simulink model "pfb_fft_vacc_cfloat_fft"  (R2016b) (continued)
== system pfb_fft_vacc_cfloat_fft/pfb/fft_wideband_real/fft_biplex_real_4x/biplex_core == (continued)
      | end
      mask Callback use_embedded:
      | % make mutually exclusive with use_hdl.
      | 
      | use_hdl = get_param(gcb, 'use_embedded');
      | if strcmp(use_hdl, 'on'),
      |     set_param_state(gcb, 'use_hdl', 'off');
      | else
      |     set_param_state(gcb, 'use_hdl', 'on');
      | end
      mask Callback hardcode_shifts:
      | hardcode_shifts = get_param(gcb, 'hardcode_shifts');
      | set_param_state(gcb, 'downshift', hardcode_shifts);
      mask Callback dsp48_adders: fft_callback_dsp48_adders(gcb);
  fft_stage_3 : Subsystem  AncestorBlock=casper_library_ffts/fft_stage_n  mask(fft_stage_n){n_inputs=4, FFTSize=10, FFTStage=3, input_bit_width=18, bin_pt_in=9, coeff_bit_width=18, async=on, add_latency=2, mult_latency=3, bram_latency=4, conv_latency=4, quantization=Round  (unbiased: +/- Inf), overflow=Wrap, delays_bram=off, coeffs_bit_limit=13, coeff_sharing=on, coeff_decimation=on, max_fanout=1, use_hdl=on, use_embedded=off, bitgrowth=off, hardcode_shifts=off, downshift=off, dsp48_adders=off}
      mask prompts: n_inputs "Number inputs"; FFTSize "Size of FFT: (2^? pts)"; FFTStage "Stage of FFT:"; input_bit_width "Input Bit Width"; bin_pt_in "Input binary point"; coeff_bit_width "Coefficient Bit Width"; async "Asynchronous operation"; add_latency "Add Latency"; mult_latency "Mult Latency"; bram_latency "BRAM Latency"; conv_latency "Convert latency"; quantization "Quantization Behavior"; overflow "Overflow Behavior"; delays_bram "Implement Delays in BRAM"; coeffs_bit_limit "Number bits above which to store stage's coefficients in BRAM (2^? bits)"; coeff_sharing "BRAM sharing in coeff storage"; coeff_decimation "Store a fraction of coeff factors where useful"; max_fanout "Maximum fanout"; use_hdl "Use behavioural HDL for mutlipliers"; use_embedded "Use embedded multipliers"; bitgrowth "Bit growth instead of shifting"; hardcode_shifts "Hardcode shift schedule"; dsp48_adders "Use DSP48s for adders"
      mask help: FFT internal block.
      mask init: same as pfb_fft_vacc_cfloat_fft/pfb/fft_wideband_real/fft_biplex_real_4x/biplex_core/fft_stage_1
      settings: AttributesFormatString=|
      | [3/10]
      | delays in slices
      | dynamic shifting
      mask TypeOptions quantization: Truncate | Round  (unbiased: +/- Inf) | Round  (unbiased: Even Values)
      mask TypeOptions overflow: Wrap | Saturate | Error
      mask Callback use_hdl:
      | % make mutually exclusive with use_embedded.
      | 
      | use_hdl = get_param(gcb, 'use_hdl');
      | if strcmp(use_hdl, 'on'),
      |     set_param_state(gcb, 'use_embedded', 'off');
      | else
      |     set_param_state(gcb, 'use_embedded', 'on');
      | end
      mask Callback use_embedded:
      | % make mutually exclusive with use_hdl.
      | 
      | use_hdl = get_param(gcb, 'use_embedded');
      | if strcmp(use_hdl, 'on'),
      |     set_param_state(gcb, 'use_hdl', 'off');
      | else
      |     set_param_state(gcb, 'use_hdl', 'on');
      | end
      mask Callback hardcode_shifts:
      | hardcode_shifts = get_param(gcb, 'hardcode_shifts');
      | set_param_state(gcb, 'downshift', hardcode_shifts);
      mask Callback dsp48_adders: fft_callback_dsp48_adders(gcb);
  fft_stage_4 : Subsystem  AncestorBlock=casper_library_ffts/fft_stage_n  mask(fft_stage_n){n_inputs=4, FFTSize=10, FFTStage=4, input_bit_width=18, bin_pt_in=9, coeff_bit_width=18, async=on, add_latency=2, mult_latency=3, bram_latency=4, conv_latency=4, quantization=Round  (unbiased: +/- Inf), overflow=Wrap, delays_bram=off, coeffs_bit_limit=13, coeff_sharing=on, coeff_decimation=on, max_fanout=1, use_hdl=on, use_embedded=off, bitgrowth=off, hardcode_shifts=off, downshift=off, dsp48_adders=off}
      mask prompts: n_inputs "Number inputs"; FFTSize "Size of FFT: (2^? pts)"; FFTStage "Stage of FFT:"; input_bit_width "Input Bit Width"; bin_pt_in "Input binary point"; coeff_bit_width "Coefficient Bit Width"; async "Asynchronous operation"; add_latency "Add Latency"; mult_latency "Mult Latency"; bram_latency "BRAM Latency"; conv_latency "Convert latency"; quantization "Quantization Behavior"; overflow "Overflow Behavior"; delays_bram "Implement Delays in BRAM"; coeffs_bit_limit "Number bits above which to store stage's coefficients in BRAM (2^? bits)"; coeff_sharing "BRAM sharing in coeff storage"; coeff_decimation "Store a fraction of coeff factors where useful"; max_fanout "Maximum fanout"; use_hdl "Use behavioural HDL for mutlipliers"; use_embedded "Use embedded multipliers"; bitgrowth "Bit growth instead of shifting"; hardcode_shifts "Hardcode shift schedule"; dsp48_adders "Use DSP48s for adders"
      mask help: FFT internal block.
      mask init: same as pfb_fft_vacc_cfloat_fft/pfb/fft_wideband_real/fft_biplex_real_4x/biplex_core/fft_stage_1
      settings: AttributesFormatString=|
      | [4/10]
      | delays in slices
      | dynamic shifting
      mask TypeOptions quantization: Truncate | Round  (unbiased: +/- Inf) | Round  (unbiased: Even Values)
      mask TypeOptions overflow: Wrap | Saturate | Error
      mask Callback use_hdl:
      | % make mutually exclusive with use_embedded.
      | 
      | use_hdl = get_param(gcb, 'use_hdl');
      | if strcmp(use_hdl, 'on'),
      |     set_param_state(gcb, 'use_embedded', 'off');
      | else
      |     set_param_state(gcb, 'use_embedded', 'on');
      | end
      mask Callback use_embedded:
      | % make mutually exclusive with use_hdl.
      | 
      | use_hdl = get_param(gcb, 'use_embedded');
      | if strcmp(use_hdl, 'on'),
      |     set_param_state(gcb, 'use_hdl', 'off');
      | else
      |     set_param_state(gcb, 'use_hdl', 'on');
      | end
      mask Callback hardcode_shifts:
      | hardcode_shifts = get_param(gcb, 'hardcode_shifts');
      | set_param_state(gcb, 'downshift', hardcode_shifts);
      mask Callback dsp48_adders: fft_callback_dsp48_adders(gcb);
  fft_stage_5 : Subsystem  AncestorBlock=casper_library_ffts/fft_stage_n  mask(fft_stage_n){n_inputs=4, FFTSize=10, FFTStage=5, input_bit_width=18, bin_pt_in=9, coeff_bit_width=18, async=on, add_latency=2, mult_latency=3, bram_latency=4, conv_latency=4, quantization=Round  (unbiased: +/- Inf), overflow=Wrap, delays_bram=off, coeffs_bit_limit=13, coeff_sharing=on, coeff_decimation=on, max_fanout=1, use_hdl=on, use_embedded=off, bitgrowth=off, hardcode_shifts=off, downshift=off, dsp48_adders=off}
      mask prompts: n_inputs "Number inputs"; FFTSize "Size of FFT: (2^? pts)"; FFTStage "Stage of FFT:"; input_bit_width "Input Bit Width"; bin_pt_in "Input binary point"; coeff_bit_width "Coefficient Bit Width"; async "Asynchronous operation"; add_latency "Add Latency"; mult_latency "Mult Latency"; bram_latency "BRAM Latency"; conv_latency "Convert latency"; quantization "Quantization Behavior"; overflow "Overflow Behavior"; delays_bram "Implement Delays in BRAM"; coeffs_bit_limit "Number bits above which to store stage's coefficients in BRAM (2^? bits)"; coeff_sharing "BRAM sharing in coeff storage"; coeff_decimation "Store a fraction of coeff factors where useful"; max_fanout "Maximum fanout"; use_hdl "Use behavioural HDL for mutlipliers"; use_embedded "Use embedded multipliers"; bitgrowth "Bit growth instead of shifting"; hardcode_shifts "Hardcode shift schedule"; dsp48_adders "Use DSP48s for adders"
      mask help: FFT internal block.
      mask init: same as pfb_fft_vacc_cfloat_fft/pfb/fft_wideband_real/fft_biplex_real_4x/biplex_core/fft_stage_1
      settings: AttributesFormatString=|
      | [5/10]
      | delays in slices
      | dynamic shifting
      mask TypeOptions quantization: Truncate | Round  (unbiased: +/- Inf) | Round  (unbiased: Even Values)
      mask TypeOptions overflow: Wrap | Saturate | Error
      mask Callback use_hdl:
      | % make mutually exclusive with use_embedded.
      | 
      | use_hdl = get_param(gcb, 'use_hdl');
      | if strcmp(use_hdl, 'on'),
      |     set_param_state(gcb, 'use_embedded', 'off');
      | else
      |     set_param_state(gcb, 'use_embedded', 'on');
      | end
      mask Callback use_embedded:
      | % make mutually exclusive with use_hdl.
      | 
      | use_hdl = get_param(gcb, 'use_embedded');
      | if strcmp(use_hdl, 'on'),
      |     set_param_state(gcb, 'use_hdl', 'off');
      | else
      |     set_param_state(gcb, 'use_hdl', 'on');
      | end
      mask Callback hardcode_shifts:
      | hardcode_shifts = get_param(gcb, 'hardcode_shifts');
      | set_param_state(gcb, 'downshift', hardcode_shifts);
      mask Callback dsp48_adders: fft_callback_dsp48_adders(gcb);
  fft_stage_6 : Subsystem  AncestorBlock=casper_library_ffts/fft_stage_n  mask(fft_stage_n){n_inputs=4, FFTSize=10, FFTStage=6, input_bit_width=18, bin_pt_in=9, coeff_bit_width=18, async=on, add_latency=2, mult_latency=3, bram_latency=4, conv_latency=4, quantization=Round  (unbiased: +/- Inf), overflow=Wrap, delays_bram=off, coeffs_bit_limit=13, coeff_sharing=on, coeff_decimation=on, max_fanout=1, use_hdl=on, use_embedded=off, bitgrowth=off, hardcode_shifts=off, downshift=off, dsp48_adders=off}
      mask prompts: n_inputs "Number inputs"; FFTSize "Size of FFT: (2^? pts)"; FFTStage "Stage of FFT:"; input_bit_width "Input Bit Width"; bin_pt_in "Input binary point"; coeff_bit_width "Coefficient Bit Width"; async "Asynchronous operation"; add_latency "Add Latency"; mult_latency "Mult Latency"; bram_latency "BRAM Latency"; conv_latency "Convert latency"; quantization "Quantization Behavior"; overflow "Overflow Behavior"; delays_bram "Implement Delays in BRAM"; coeffs_bit_limit "Number bits above which to store stage's coefficients in BRAM (2^? bits)"; coeff_sharing "BRAM sharing in coeff storage"; coeff_decimation "Store a fraction of coeff factors where useful"; max_fanout "Maximum fanout"; use_hdl "Use behavioural HDL for mutlipliers"; use_embedded "Use embedded multipliers"; bitgrowth "Bit growth instead of shifting"; hardcode_shifts "Hardcode shift schedule"; dsp48_adders "Use DSP48s for adders"
      mask help: FFT internal block.
      mask init: same as pfb_fft_vacc_cfloat_fft/pfb/fft_wideband_real/fft_biplex_real_4x/biplex_core/fft_stage_1
      settings: AttributesFormatString=|
      | [6/10]
      | delays in slices
      | dynamic shifting
      mask TypeOptions quantization: Truncate | Round  (unbiased: +/- Inf) | Round  (unbiased: Even Values)
      mask TypeOptions overflow: Wrap | Saturate | Error
      mask Callback use_hdl:
      | % make mutually exclusive with use_embedded.
      | 
      | use_hdl = get_param(gcb, 'use_hdl');
      | if strcmp(use_hdl, 'on'),
      |     set_param_state(gcb, 'use_embedded', 'off');
      | else
      |     set_param_state(gcb, 'use_embedded', 'on');
      | end
      mask Callback use_embedded:
      | % make mutually exclusive with use_hdl.
      | 
      | use_hdl = get_param(gcb, 'use_embedded');
      | if strcmp(use_hdl, 'on'),
      |     set_param_state(gcb, 'use_hdl', 'off');
      | else
      |     set_param_state(gcb, 'use_hdl', 'on');
      | end
      mask Callback hardcode_shifts:
      | hardcode_shifts = get_param(gcb, 'hardcode_shifts');
      | set_param_state(gcb, 'downshift', hardcode_shifts);
      mask Callback dsp48_adders: fft_callback_dsp48_adders(gcb);
  fft_stage_7 : Subsystem  AncestorBlock=casper_library_ffts/fft_stage_n  mask(fft_stage_n){n_inputs=4, FFTSize=10, FFTStage=7, input_bit_width=18, bin_pt_in=9, coeff_bit_width=18, async=on, add_latency=2, mult_latency=3, bram_latency=4, conv_latency=4, quantization=Round  (unbiased: +/- Inf), overflow=Wrap, delays_bram=off, coeffs_bit_limit=13, coeff_sharing=on, coeff_decimation=on, max_fanout=1, use_hdl=on, use_embedded=off, bitgrowth=off, hardcode_shifts=off, downshift=off, dsp48_adders=off}
      mask prompts: n_inputs "Number inputs"; FFTSize "Size of FFT: (2^? pts)"; FFTStage "Stage of FFT:"; input_bit_width "Input Bit Width"; bin_pt_in "Input binary point"; coeff_bit_width "Coefficient Bit Width"; async "Asynchronous operation"; add_latency "Add Latency"; mult_latency "Mult Latency"; bram_latency "BRAM Latency"; conv_latency "Convert latency"; quantization "Quantization Behavior"; overflow "Overflow Behavior"; delays_bram "Implement Delays in BRAM"; coeffs_bit_limit "Number bits above which to store stage's coefficients in BRAM (2^? bits)"; coeff_sharing "BRAM sharing in coeff storage"; coeff_decimation "Store a fraction of coeff factors where useful"; max_fanout "Maximum fanout"; use_hdl "Use behavioural HDL for mutlipliers"; use_embedded "Use embedded multipliers"; bitgrowth "Bit growth instead of shifting"; hardcode_shifts "Hardcode shift schedule"; dsp48_adders "Use DSP48s for adders"
      mask help: FFT internal block.
      mask init: same as pfb_fft_vacc_cfloat_fft/pfb/fft_wideband_real/fft_biplex_real_4x/biplex_core/fft_stage_1
      settings: AttributesFormatString=|
      | [7/10]
      | delays in slices
      | dynamic shifting
      mask TypeOptions quantization: Truncate | Round  (unbiased: +/- Inf) | Round  (unbiased: Even Values)
      mask TypeOptions overflow: Wrap | Saturate | Error
      mask Callback use_hdl:
      | % make mutually exclusive with use_embedded.
      | 
      | use_hdl = get_param(gcb, 'use_hdl');
      | if strcmp(use_hdl, 'on'),
      |     set_param_state(gcb, 'use_embedded', 'off');
      | else
      |     set_param_state(gcb, 'use_embedded', 'on');
      | end
      mask Callback use_embedded:
      | % make mutually exclusive with use_hdl.
      | 
      | use_hdl = get_param(gcb, 'use_embedded');
      | if strcmp(use_hdl, 'on'),
      |     set_param_state(gcb, 'use_hdl', 'off');
      | else
      |     set_param_state(gcb, 'use_hdl', 'on');
      | end
      mask Callback hardcode_shifts:
      | hardcode_shifts = get_param(gcb, 'hardcode_shifts');
      | set_param_state(gcb, 'downshift', hardcode_shifts);
      mask Callback dsp48_adders: fft_callback_dsp48_adders(gcb);
  fft_stage_8 : Subsystem  AncestorBlock=casper_library_ffts/fft_stage_n  mask(fft_stage_n){n_inputs=4, FFTSize=10, FFTStage=8, input_bit_width=18, bin_pt_in=9, coeff_bit_width=18, async=on, add_latency=2, mult_latency=3, bram_latency=4, conv_latency=4, quantization=Round  (unbiased: +/- Inf), overflow=Wrap, delays_bram=off, coeffs_bit_limit=13, coeff_sharing=on, coeff_decimation=on, max_fanout=1, use_hdl=on, use_embedded=off, bitgrowth=off, hardcode_shifts=off, downshift=off, dsp48_adders=off}
      mask prompts: n_inputs "Number inputs"; FFTSize "Size of FFT: (2^? pts)"; FFTStage "Stage of FFT:"; input_bit_width "Input Bit Width"; bin_pt_in "Input binary point"; coeff_bit_width "Coefficient Bit Width"; async "Asynchronous operation"; add_latency "Add Latency"; mult_latency "Mult Latency"; bram_latency "BRAM Latency"; conv_latency "Convert latency"; quantization "Quantization Behavior"; overflow "Overflow Behavior"; delays_bram "Implement Delays in BRAM"; coeffs_bit_limit "Number bits above which to store stage's coefficients in BRAM (2^? bits)"; coeff_sharing "BRAM sharing in coeff storage"; coeff_decimation "Store a fraction of coeff factors where useful"; max_fanout "Maximum fanout"; use_hdl "Use behavioural HDL for mutlipliers"; use_embedded "Use embedded multipliers"; bitgrowth "Bit growth instead of shifting"; hardcode_shifts "Hardcode shift schedule"; dsp48_adders "Use DSP48s for adders"
      mask help: FFT internal block.
      mask init: same as pfb_fft_vacc_cfloat_fft/pfb/fft_wideband_real/fft_biplex_real_4x/biplex_core/fft_stage_1
      settings: AttributesFormatString=|
      | [8/10]
      | delays in slices
      | dynamic shifting
      mask TypeOptions quantization: Truncate | Round  (unbiased: +/- Inf) | Round  (unbiased: Even Values)
      mask TypeOptions overflow: Wrap | Saturate | Error
      mask Callback use_hdl:
      | % make mutually exclusive with use_embedded.
      | 
      | use_hdl = get_param(gcb, 'use_hdl');
      | if strcmp(use_hdl, 'on'),
      |     set_param_state(gcb, 'use_embedded', 'off');
      | else
      |     set_param_state(gcb, 'use_embedded', 'on');
      | end
      mask Callback use_embedded:
      | % make mutually exclusive with use_hdl.
      | 
      | use_hdl = get_param(gcb, 'use_embedded');
      | if strcmp(use_hdl, 'on'),
      |     set_param_state(gcb, 'use_hdl', 'off');
      | else
      |     set_param_state(gcb, 'use_hdl', 'on');
      | end
      mask Callback hardcode_shifts:
      | hardcode_shifts = get_param(gcb, 'hardcode_shifts');
      | set_param_state(gcb, 'downshift', hardcode_shifts);
      mask Callback dsp48_adders: fft_callback_dsp48_adders(gcb);
  fft_stage_9 : Subsystem  AncestorBlock=casper_library_ffts/fft_stage_n  mask(fft_stage_n){n_inputs=4, FFTSize=10, FFTStage=9, input_bit_width=18, bin_pt_in=9, coeff_bit_width=18, async=on, add_latency=2, mult_latency=3, bram_latency=4, conv_latency=4, quantization=Round  (unbiased: +/- Inf), overflow=Wrap, delays_bram=off, coeffs_bit_limit=13, coeff_sharing=on, coeff_decimation=on, max_fanout=1, use_hdl=on, use_embedded=off, bitgrowth=off, hardcode_shifts=off, downshift=off, dsp48_adders=off}
      mask prompts: n_inputs "Number inputs"; FFTSize "Size of FFT: (2^? pts)"; FFTStage "Stage of FFT:"; input_bit_width "Input Bit Width"; bin_pt_in "Input binary point"; coeff_bit_width "Coefficient Bit Width"; async "Asynchronous operation"; add_latency "Add Latency"; mult_latency "Mult Latency"; bram_latency "BRAM Latency"; conv_latency "Convert latency"; quantization "Quantization Behavior"; overflow "Overflow Behavior"; delays_bram "Implement Delays in BRAM"; coeffs_bit_limit "Number bits above which to store stage's coefficients in BRAM (2^? bits)"; coeff_sharing "BRAM sharing in coeff storage"; coeff_decimation "Store a fraction of coeff factors where useful"; max_fanout "Maximum fanout"; use_hdl "Use behavioural HDL for mutlipliers"; use_embedded "Use embedded multipliers"; bitgrowth "Bit growth instead of shifting"; hardcode_shifts "Hardcode shift schedule"; dsp48_adders "Use DSP48s for adders"
      mask help: FFT internal block.
      mask init: same as pfb_fft_vacc_cfloat_fft/pfb/fft_wideband_real/fft_biplex_real_4x/biplex_core/fft_stage_1
      settings: AttributesFormatString=|
      | [9/10]
      | delays in slices
      | dynamic shifting
      mask TypeOptions quantization: Truncate | Round  (unbiased: +/- Inf) | Round  (unbiased: Even Values)
      mask TypeOptions overflow: Wrap | Saturate | Error
      mask Callback use_hdl:
      | % make mutually exclusive with use_embedded.
      | 
      | use_hdl = get_param(gcb, 'use_hdl');
      | if strcmp(use_hdl, 'on'),
      |     set_param_state(gcb, 'use_embedded', 'off');
      | else
      |     set_param_state(gcb, 'use_embedded', 'on');
      | end
      mask Callback use_embedded:
      | % make mutually exclusive with use_hdl.
      | 
      | use_hdl = get_param(gcb, 'use_embedded');
      | if strcmp(use_hdl, 'on'),
      |     set_param_state(gcb, 'use_hdl', 'off');
      | else
      |     set_param_state(gcb, 'use_hdl', 'on');
      | end
      mask Callback hardcode_shifts:
      | hardcode_shifts = get_param(gcb, 'hardcode_shifts');
      | set_param_state(gcb, 'downshift', hardcode_shifts);
      mask Callback dsp48_adders: fft_callback_dsp48_adders(gcb);
  sync_out : Outport
  out1 : Outport  Port=2
  out2 : Outport  Port=3
  of : Outport  Port=4
  dvalid : Outport  Port=5
  wires:
  pol1/1 -> fft_stage_1/1(in1)
  pol2/1 -> fft_stage_1/2(in2)
  Constant/1 -> fft_stage_1/3(of_in)
  sync/1 -> fft_stage_1/4(sync)
  fft_stage_10/1(out1) -> bus_expand10/1(bus_in), out1/1
  fft_stage_10/2(out2) -> out2/1
  fft_stage_10/3(of) -> of/1
  fft_stage_10/4(sync_out) -> sync_out/1
  en/1 -> fft_stage_1/6(en)
  fft_stage_10/5(dvalid) -> dvalid/1
  fft_stage_1/2(out2) -> fft_stage_2/2(in2)
  fft_stage_1/3(of) -> fft_stage_2/3(of_in)
  fft_stage_1/4(sync_out) -> fft_stage_2/4(sync)
  shift/1 -> fft_stage_2/5(shift), fft_stage_10/5(shift), fft_stage_9/5(shift), fft_stage_8/5(shift), fft_stage_7/5(shift), fft_stage_6/5(shift), fft_stage_5/5(shift), fft_stage_4/5(shift), fft_stage_3/5(shift), fft_stage_1/5(shift)
  fft_stage_1/5(dvalid) -> fft_stage_2/6(en)
  fft_stage_2/1(out1) -> bus_expand2/1(bus_in), fft_stage_3/1(in1)
  fft_stage_2/2(out2) -> fft_stage_3/2(in2)
  fft_stage_2/3(of) -> fft_stage_3/3(of_in)
  fft_stage_2/4(sync_out) -> fft_stage_3/4(sync)
  fft_stage_2/5(dvalid) -> fft_stage_3/6(en)
  fft_stage_3/1(out1) -> bus_expand3/1(bus_in), fft_stage_4/1(in1)
  fft_stage_3/3(of) -> fft_stage_4/3(of_in)
  fft_stage_3/4(sync_out) -> fft_stage_4/4(sync)
  fft_stage_3/5(dvalid) -> fft_stage_4/6(en)
  fft_stage_3/2(out2) -> fft_stage_4/2(in2)
  fft_stage_4/1(out1) -> bus_expand4/1(bus_in), fft_stage_5/1(in1)
  fft_stage_4/3(of) -> fft_stage_5/3(of_in)
  fft_stage_4/2(out2) -> fft_stage_5/2(in2)
  fft_stage_4/5(dvalid) -> fft_stage_5/6(en)
  fft_stage_4/4(sync_out) -> fft_stage_5/4(sync)
  fft_stage_5/2(out2) -> fft_stage_6/2(in2)
  fft_stage_5/3(of) -> fft_stage_6/3(of_in)
  fft_stage_5/4(sync_out) -> fft_stage_6/4(sync)
  fft_stage_5/1(out1) -> bus_expand5/1(bus_in), fft_stage_6/1(in1)
  fft_stage_5/5(dvalid) -> fft_stage_6/6(en)
  fft_stage_6/5(dvalid) -> fft_stage_7/6(en)
  fft_stage_6/1(out1) -> bus_expand6/1(bus_in), fft_stage_7/1(in1)
  fft_stage_6/3(of) -> fft_stage_7/3(of_in)
  fft_stage_6/4(sync_out) -> fft_stage_7/4(sync)
  fft_stage_6/2(out2) -> fft_stage_7/2(in2)
  fft_stage_7/4(sync_out) -> fft_stage_8/4(sync)
  fft_stage_7/3(of) -> fft_stage_8/3(of_in)
  fft_stage_7/5(dvalid) -> fft_stage_8/6(en)
  fft_stage_7/1(out1) -> bus_expand7/1(bus_in), fft_stage_8/1(in1)
  fft_stage_7/2(out2) -> fft_stage_8/2(in2)
  fft_stage_8/5(dvalid) -> fft_stage_9/6(en)
  fft_stage_8/4(sync_out) -> fft_stage_9/4(sync)
  fft_stage_8/2(out2) -> fft_stage_9/2(in2)
  fft_stage_8/3(of) -> fft_stage_9/3(of_in)
  fft_stage_8/1(out1) -> bus_expand8/1(bus_in), fft_stage_9/1(in1)
  fft_stage_9/1(out1) -> bus_expand9/1(bus_in), fft_stage_10/1(in1)
  fft_stage_9/5(dvalid) -> fft_stage_10/6(en)
  fft_stage_9/4(sync_out) -> fft_stage_10/4(sync)
  fft_stage_9/2(out2) -> fft_stage_10/2(in2)
  fft_stage_9/3(of) -> fft_stage_10/3(of_in)
  fft_stage_1/1(out1) -> bus_expand1/1(bus_in), fft_stage_2/1(in1)
  Gateway Out6/1 -> To Workspace5/1
  Reinterpret4/1 -> Gateway Out6/1
  bus_expand1/1(msb_out8) -> Reinterpret4/1
  Gateway Out1/1 -> To Workspace1/1
  Reinterpret1/1 -> Gateway Out1/1
  bus_expand1/2(out7) -> Reinterpret1/1
  Gateway Out2/1 -> To Workspace2/1
  Reinterpret2/1 -> Gateway Out2/1
  bus_expand1/3(out6) -> Reinterpret2/1
  Gateway Out3/1 -> To Workspace3/1
  Reinterpret3/1 -> Gateway Out3/1
  bus_expand1/4(out5) -> Reinterpret3/1
  Gateway Out4/1 -> To Workspace4/1
  Reinterpret5/1 -> Gateway Out4/1
  bus_expand1/5(out4) -> Reinterpret5/1
  Gateway Out5/1 -> To Workspace6/1
  Reinterpret6/1 -> Gateway Out5/1
  bus_expand1/6(out3) -> Reinterpret6/1
  Gateway Out7/1 -> To Workspace7/1
  Reinterpret7/1 -> Gateway Out7/1
  bus_expand1/7(out2) -> Reinterpret7/1
  Gateway Out8/1 -> To Workspace8/1
  Reinterpret8/1 -> Gateway Out8/1
  bus_expand1/8(lsb_out1) -> Reinterpret8/1
  Gateway Out14/1 -> To Workspace13/1
  Reinterpret12/1 -> Gateway Out14/1
  bus_expand2/1(msb_out8) -> Reinterpret12/1
  Gateway Out9/1 -> To Workspace9/1
  Reinterpret9/1 -> Gateway Out9/1
  bus_expand2/2(out7) -> Reinterpret9/1
  Gateway Out10/1 -> To Workspace10/1
  Reinterpret10/1 -> Gateway Out10/1
  bus_expand2/3(out6) -> Reinterpret10/1
  Gateway Out11/1 -> To Workspace11/1
  Reinterpret11/1 -> Gateway Out11/1
  bus_expand2/4(out5) -> Reinterpret11/1
  Gateway Out12/1 -> To Workspace12/1
  Reinterpret13/1 -> Gateway Out12/1
  bus_expand2/5(out4) -> Reinterpret13/1
  Gateway Out13/1 -> To Workspace14/1
  Reinterpret14/1 -> Gateway Out13/1
  bus_expand2/6(out3) -> Reinterpret14/1
  Gateway Out15/1 -> To Workspace15/1
  Reinterpret15/1 -> Gateway Out15/1
  bus_expand2/7(out2) -> Reinterpret15/1
  Gateway Out16/1 -> To Workspace16/1
  Reinterpret16/1 -> Gateway Out16/1
  bus_expand2/8(lsb_out1) -> Reinterpret16/1
  Gateway Out21/1 -> To Workspace20/1
  Reinterpret19/1 -> Gateway Out21/1
  bus_expand3/1(msb_out8) -> Reinterpret19/1
  Gateway Out24/1 -> To Workspace24/1
  Reinterpret24/1 -> Gateway Out24/1
  bus_expand3/2(out7) -> Reinterpret24/1
  Gateway Out17/1 -> To Workspace17/1
  Reinterpret17/1 -> Gateway Out17/1
  bus_expand3/3(out6) -> Reinterpret17/1
  Gateway Out18/1 -> To Workspace18/1
  Reinterpret18/1 -> Gateway Out18/1
  bus_expand3/4(out5) -> Reinterpret18/1
  Gateway Out19/1 -> To Workspace19/1
  Reinterpret20/1 -> Gateway Out19/1
  bus_expand3/5(out4) -> Reinterpret20/1
  Gateway Out20/1 -> To Workspace21/1
  Reinterpret21/1 -> Gateway Out20/1
  bus_expand3/6(out3) -> Reinterpret21/1
  Gateway Out22/1 -> To Workspace22/1
  Reinterpret22/1 -> Gateway Out22/1
  bus_expand3/7(out2) -> Reinterpret22/1
  Gateway Out23/1 -> To Workspace23/1
  Reinterpret23/1 -> Gateway Out23/1
  bus_expand3/8(lsb_out1) -> Reinterpret23/1
  Gateway Out29/1 -> To Workspace28/1
  Reinterpret27/1 -> Gateway Out29/1
  bus_expand4/1(msb_out8) -> Reinterpret27/1
  Gateway Out32/1 -> To Workspace32/1
  Reinterpret32/1 -> Gateway Out32/1
  bus_expand4/2(out7) -> Reinterpret32/1
  Gateway Out25/1 -> To Workspace25/1
  Reinterpret25/1 -> Gateway Out25/1
  bus_expand4/3(out6) -> Reinterpret25/1
  Gateway Out26/1 -> To Workspace26/1
  Reinterpret26/1 -> Gateway Out26/1
  bus_expand4/4(out5) -> Reinterpret26/1
  Gateway Out27/1 -> To Workspace27/1
  Reinterpret28/1 -> Gateway Out27/1
  bus_expand4/5(out4) -> Reinterpret28/1
  Gateway Out28/1 -> To Workspace29/1
  Reinterpret29/1 -> Gateway Out28/1
  bus_expand4/6(out3) -> Reinterpret29/1
  Gateway Out30/1 -> To Workspace30/1
  Reinterpret30/1 -> Gateway Out30/1
  bus_expand4/7(out2) -> Reinterpret30/1
  Gateway Out31/1 -> To Workspace31/1
  Reinterpret31/1 -> Gateway Out31/1
  bus_expand4/8(lsb_out1) -> Reinterpret31/1
  Gateway Out37/1 -> To Workspace36/1
  Reinterpret35/1 -> Gateway Out37/1
  bus_expand5/1(msb_out8) -> Reinterpret35/1
  Gateway Out40/1 -> To Workspace40/1
  Reinterpret40/1 -> Gateway Out40/1
  bus_expand5/2(out7) -> Reinterpret40/1
  Gateway Out33/1 -> To Workspace33/1
  Reinterpret33/1 -> Gateway Out33/1
  bus_expand5/3(out6) -> Reinterpret33/1
  Gateway Out34/1 -> To Workspace34/1
  Reinterpret34/1 -> Gateway Out34/1
  bus_expand5/4(out5) -> Reinterpret34/1
  Gateway Out35/1 -> To Workspace35/1
  Reinterpret36/1 -> Gateway Out35/1
  bus_expand5/5(out4) -> Reinterpret36/1
  Gateway Out36/1 -> To Workspace37/1
  Reinterpret37/1 -> Gateway Out36/1
  bus_expand5/6(out3) -> Reinterpret37/1
  Gateway Out38/1 -> To Workspace38/1
  Reinterpret38/1 -> Gateway Out38/1
  bus_expand5/7(out2) -> Reinterpret38/1
  Gateway Out39/1 -> To Workspace39/1
  Reinterpret39/1 -> Gateway Out39/1
  bus_expand5/8(lsb_out1) -> Reinterpret39/1
  Gateway Out45/1 -> To Workspace44/1
  Reinterpret43/1 -> Gateway Out45/1
  bus_expand6/1(msb_out8) -> Reinterpret43/1
  Gateway Out48/1 -> To Workspace48/1
  Reinterpret48/1 -> Gateway Out48/1
  bus_expand6/2(out7) -> Reinterpret48/1
  Gateway Out41/1 -> To Workspace41/1
  Reinterpret41/1 -> Gateway Out41/1
  bus_expand6/3(out6) -> Reinterpret41/1
  Gateway Out42/1 -> To Workspace42/1
  Reinterpret42/1 -> Gateway Out42/1
  bus_expand6/4(out5) -> Reinterpret42/1
  Gateway Out43/1 -> To Workspace43/1
  Reinterpret44/1 -> Gateway Out43/1
  bus_expand6/5(out4) -> Reinterpret44/1
  Gateway Out44/1 -> To Workspace45/1
  Reinterpret45/1 -> Gateway Out44/1
  bus_expand6/6(out3) -> Reinterpret45/1
  Gateway Out46/1 -> To Workspace46/1
  Reinterpret46/1 -> Gateway Out46/1
  bus_expand6/7(out2) -> Reinterpret46/1
  Gateway Out47/1 -> To Workspace47/1
  Reinterpret47/1 -> Gateway Out47/1
  bus_expand6/8(lsb_out1) -> Reinterpret47/1
  Gateway Out53/1 -> To Workspace52/1
  Reinterpret51/1 -> Gateway Out53/1
  bus_expand7/1(msb_out8) -> Reinterpret51/1
  Gateway Out56/1 -> To Workspace56/1
  Reinterpret56/1 -> Gateway Out56/1
  bus_expand7/2(out7) -> Reinterpret56/1
  Gateway Out49/1 -> To Workspace49/1
  Reinterpret49/1 -> Gateway Out49/1
  bus_expand7/3(out6) -> Reinterpret49/1
  Gateway Out50/1 -> To Workspace50/1
  Reinterpret50/1 -> Gateway Out50/1
  bus_expand7/4(out5) -> Reinterpret50/1
  Gateway Out51/1 -> To Workspace51/1
  Reinterpret52/1 -> Gateway Out51/1
  bus_expand7/5(out4) -> Reinterpret52/1
  Gateway Out52/1 -> To Workspace53/1
  Reinterpret53/1 -> Gateway Out52/1
  bus_expand7/6(out3) -> Reinterpret53/1
  Gateway Out54/1 -> To Workspace54/1
  Reinterpret54/1 -> Gateway Out54/1
  bus_expand7/7(out2) -> Reinterpret54/1
  Gateway Out55/1 -> To Workspace55/1
  Reinterpret55/1 -> Gateway Out55/1
  bus_expand7/8(lsb_out1) -> Reinterpret55/1
  Gateway Out61/1 -> To Workspace60/1
  Reinterpret59/1 -> Gateway Out61/1
  bus_expand8/1(msb_out8) -> Reinterpret59/1
  Gateway Out64/1 -> To Workspace64/1
  Reinterpret64/1 -> Gateway Out64/1
  bus_expand8/2(out7) -> Reinterpret64/1
  Gateway Out57/1 -> To Workspace57/1
  Reinterpret57/1 -> Gateway Out57/1
  bus_expand8/3(out6) -> Reinterpret57/1
  Gateway Out58/1 -> To Workspace58/1
  Reinterpret58/1 -> Gateway Out58/1
  bus_expand8/4(out5) -> Reinterpret58/1
  Gateway Out59/1 -> To Workspace59/1
  Reinterpret60/1 -> Gateway Out59/1
  bus_expand8/5(out4) -> Reinterpret60/1
  Gateway Out60/1 -> To Workspace61/1
  Reinterpret61/1 -> Gateway Out60/1
  bus_expand8/6(out3) -> Reinterpret61/1
  Gateway Out62/1 -> To Workspace62/1
  Reinterpret62/1 -> Gateway Out62/1
  bus_expand8/7(out2) -> Reinterpret62/1
  Gateway Out63/1 -> To Workspace63/1
  Reinterpret63/1 -> Gateway Out63/1
  bus_expand8/8(lsb_out1) -> Reinterpret63/1
  Gateway Out69/1 -> To Workspace68/1
  Reinterpret67/1 -> Gateway Out69/1
  bus_expand9/1(msb_out8) -> Reinterpret67/1
  Gateway Out72/1 -> To Workspace72/1
  Reinterpret72/1 -> Gateway Out72/1
  bus_expand9/2(out7) -> Reinterpret72/1
  Gateway Out65/1 -> To Workspace65/1
  Reinterpret65/1 -> Gateway Out65/1
  bus_expand9/3(out6) -> Reinterpret65/1
  Gateway Out66/1 -> To Workspace66/1
  Reinterpret66/1 -> Gateway Out66/1
  bus_expand9/4(out5) -> Reinterpret66/1
  Gateway Out67/1 -> To Workspace67/1
  Reinterpret68/1 -> Gateway Out67/1
  bus_expand9/5(out4) -> Reinterpret68/1
  Gateway Out68/1 -> To Workspace69/1
  Reinterpret69/1 -> Gateway Out68/1
  bus_expand9/6(out3) -> Reinterpret69/1
  Gateway Out70/1 -> To Workspace70/1
  Reinterpret70/1 -> Gateway Out70/1
  bus_expand9/7(out2) -> Reinterpret70/1
  Gateway Out71/1 -> To Workspace71/1
  Reinterpret71/1 -> Gateway Out71/1
  bus_expand9/8(lsb_out1) -> Reinterpret71/1
  Gateway Out77/1 -> To Workspace76/1
  Reinterpret75/1 -> Gateway Out77/1
  bus_expand10/1(msb_out8) -> Reinterpret75/1
  Gateway Out80/1 -> To Workspace80/1
  Reinterpret80/1 -> Gateway Out80/1
  bus_expand10/2(out7) -> Reinterpret80/1
  Gateway Out73/1 -> To Workspace73/1
  Reinterpret73/1 -> Gateway Out73/1
  bus_expand10/3(out6) -> Reinterpret73/1
  Gateway Out74/1 -> To Workspace74/1
  Reinterpret74/1 -> Gateway Out74/1
  bus_expand10/4(out5) -> Reinterpret74/1
  Gateway Out75/1 -> To Workspace75/1
  Reinterpret76/1 -> Gateway Out75/1
  bus_expand10/5(out4) -> Reinterpret76/1
  Gateway Out76/1 -> To Workspace77/1
  Reinterpret77/1 -> Gateway Out76/1
  bus_expand10/6(out3) -> Reinterpret77/1
  Gateway Out78/1 -> To Workspace78/1
  Reinterpret78/1 -> Gateway Out78/1
  bus_expand10/7(out2) -> Reinterpret78/1
  Gateway Out79/1 -> To Workspace79/1
  Reinterpret79/1 -> Gateway Out79/1
  bus_expand10/8(lsb_out1) -> Reinterpret79/1
